simulink model "ofdm_txrx_supermimo"  (Simulink 7.1)
settings: Solver=VariableStepDiscrete  SolverMode=SingleTasking  StartTime=0.0  StopTime=simOnly_simLength  RelTol=1e-3
PostLoadFcn:
  | ofdm_rx_supermimo_init
InitFcn:
  | ofdm_rx_supermimo_init
StartFcn:
  | ofdm_rx_supermimo_init
values from neighbouring files (symbols this model uses):
  Action0_Config = 0* 31*2^18 + 0*2^24 + PHY_AUTORESPONSE_REQ_GOODHDR + PHY_AUTORESPONSE_REQ_GOODPKT + PHY_AUTORESPONSE_REQ_FLAGA    [ofdm_tx_supermimo_init.m]
  Action1_Config = 0* 62*2^18 + PHY_AUTORESPONSE_REQ_GOODHDR + PHY_AUTORESPONSE_REQ_GOODPKT    [ofdm_tx_supermimo_init.m]
  Action2_Config = 0    [ofdm_tx_supermimo_init.m]
  Action3_Config = 0    [ofdm_tx_supermimo_init.m]
  Action4_Config = 0    [ofdm_tx_supermimo_init.m]
  Action5_Config = 0    [ofdm_tx_supermimo_init.m]
  ActionID_AFTransmit = 31    [ofdm_tx_supermimo_init.m]
  ActionID_Disabled = 0    [ofdm_tx_supermimo_init.m]
  ActionID_SetFlagA = 62    [ofdm_tx_supermimo_init.m]
  ActionID_SetFlagB = 61    [ofdm_tx_supermimo_init.m]
  BER_RAM_init_size = 2048    [ofdm_tx_supermimo_init.m]
  CPLength = 16    [ofdm_tx_supermimo_init.m]
  longCorr_coef_i = [-4*real(LTS) 0]    [ofdm_rx_supermimo_init.m]
  longCorr_coef_q = [4*imag(LTS) 0]    [ofdm_rx_supermimo_init.m]
  Match0_Config = 0    [ofdm_tx_supermimo_init.m]
  Match1_Config = 0    [ofdm_tx_supermimo_init.m]
  Match2_Config = 0    [ofdm_tx_supermimo_init.m]
  Match3_Config = 0    [ofdm_tx_supermimo_init.m]
  Match4_Config = 0    [ofdm_tx_supermimo_init.m]
  Match5_Config = 0    [ofdm_tx_supermimo_init.m]
  Match6_Config = 0    [ofdm_tx_supermimo_init.m]
  Match7_Config = 0    [ofdm_tx_supermimo_init.m]
  max_CP_length = 16    [ofdm_tx_supermimo_init.m]
  max_num_baseRateSymbols = 31    [ofdm_tx_supermimo_init.m]
  max_num_subcarriers = 64    [ofdm_tx_supermimo_init.m]
  max_num_trainingSymbols = 15    [ofdm_tx_supermimo_init.m]
  max_numBytes = 16384    [ofdm_tx_supermimo_init.m]
  max_OFDM_symbols = 511    [ofdm_tx_supermimo_init.m]
  modConstellation_qam16 = .75*[1 3 -1 -3]./3    [ofdm_tx_supermimo_init.m]
  modConstellation_qam64 = 0.875*[3 1 5 7 -3 -1 -5 -7]./7    [ofdm_tx_supermimo_init.m]
  modConstellation_qpsk = [1 -1]./sqrt(2)    [ofdm_tx_supermimo_init.m]
  numBaseRateSymbols = ceil(numHeaderBytes / numBytes_BaseRateOFDMSymbol)    [ofdm_tx_supermimo_init.m]
  numSubcarriers = 64    [ofdm_tx_supermimo_init.m]
  numTrainingSymbols = 2    [ofdm_tx_supermimo_init.m]
  pilot_indicies = 7 + ( (64-21) * 2^8) + ( (64-7) * 2^16) + (21 * 2^24)    (= 356068103)    [ofdm_tx_supermimo_init.m]
  pilotValues = hex2dec('A57D') + (2^16 * hex2dec('5A82'))    [ofdm_tx_supermimo_init.m]
  pkt_crossCorr_thresh = 0.7    [ofdm_rx_supermimo_init.m]
  pkt_energy_thresh = 0    [ofdm_rx_supermimo_init.m]
  pktByteNums = numHeaderBytes +  				byteIndex_numPayloadBytes(1)*2^8 +  				byteIndex_numPayloadBytes(2)*2^16 +  				byteIndex_simpleDynModMasks * 2^24    [ofdm_rx_supermimo_init.m]
  PktDet_Delay = 58+32 +      Symbol_Timing_Offset*2^7 +      0*2^16 +      (117* 2^24)    (= 1962935642)    [ofdm_rx_supermimo_init.m]
  pktTiming_count_payloadStart = pktTiming_count_lsStart + pktTiming_ls_num*pktTiming_ls_length    [ofdm_rx_supermimo_init.m]
  preamble = preamble_scale*[shortsyms_10 longsyms_2]    [ofdm_tx_supermimo_init.m]
  preamble_ROM = preamble_I_ROM + preamble_Q_ROM*2^16    [ofdm_tx_supermimo_init.m]
  RAM_init_size = max_numBytes    (= 16384)    [ofdm_tx_supermimo_init.m]
  reg_AF_Tx_Blanking = (320+80) + ( (320+80+79)*2^16)    (= 31392144)    [ofdm_rx_supermimo_init.m]
  reg_AF_Tx_Scaling = 2944    [ofdm_rx_supermimo_init.m]
  reg_Interrupt_PktBuf_Control = reg_InterruptControl +  	1 * 2^16 +  	0 * 2^24    (= 65656)    [ofdm_rx_supermimo_init.m]
  reg_PilotCalcParams = (4 * 2^16) + (minPilotChanMag)    (= 262184.96)    [ofdm_rx_supermimo_init.m]
  regRx_chanEst_minMag = ((0.5)*2^15) + ((0.5 * 2^15)*2^16)    (= 1073758208)    [ofdm_rx_supermimo_init.m]
  regRx_coarseCalcCorrection = round((1.3e-3 - 1.2982e-3)*2^32)    [ofdm_rx_supermimo_init.m]
  regRx_pilotCalcCorrection = round(1.00115 * 2^31)    [ofdm_rx_supermimo_init.m]
  regRx_preCFOoptions = 0 * 2^0 +  	1 * 2^1 +  	0    (= 2)    [ofdm_rx_supermimo_init.m]
  rx_controlBits = 1 * 2  		 + 1 * 4  		 + 0 * 8  		 + 1 * 16 * rx_SISO_Mode  		 + 1 * 32  		 + 1 * 64  		 + 1 * 128  		 + 1 * 256  		 + 1 * 512  		 + 1 * 1024  		 + 1 * 4096  		 + 0 * 16384  		 + 0 * 32768  		 + 1 * 65536  		 + 0 * 131072  		 + 0 * 262144  		 + 1 * 524288  		 + 1 * 1048576 * tx_Alamouti_Mode  		 + 0 * 2097152           + 1 * 33554432  		 + 0 * 4194304  		 + 0 * 8388608  		 + 0 * 16777216  		 + 1 *  ...(+49 chars)    [ofdm_rx_supermimo_init.m]
  Rx_PktDet_LongCorr_Params = LongCorrCounterLoad + 0*2^8 + 0*2^16 + 255*2^24    (= 4278190325)    [ofdm_rx_supermimo_init.m]
  Rx_PktDet_LongCorrThresholds = 8000    [ofdm_rx_supermimo_init.m]
  rx_postEq_scaling = round(rxScaling*2^11) * (1 + 2^16)    [ofdm_rx_supermimo_init.m]
  RxDataScrambling_Seq = [40 198 78 63 82 173 102 245 48 111 172 115 147 230 216 93 72 65 62 2 205 242 122 90 128 83 105 97 73 10 5 252]    [ofdm_tx_supermimo_init.m]
  RxReg_FixedPktLen = 0    [ofdm_rx_supermimo_init.m]
  shortSymbol_time = shortSymbol_time(1:16).'    [ofdm_tx_supermimo_init.m]
  simOnly_numSamples = length(preamble)+( (numSubcarriers+CPLength)*(numTrainingSymbols + numBaseRateSymbols + numFullRateSymbols/2) )    [ofdm_tx_supermimo_init.m]
  train = [train train]    [ofdm_tx_supermimo_init.m]
  tx_controlBits = (antB_preambleShift * 2^4) + (2^1 * tx_Alamouti_Mode) +  	(2^0 * tx_SISO_Mode) + 4*0 + 8*1 + 0*256 + 512*0 + 1024*0 +  	0*hex2dec('1000') + 0*hex2dec('2000') + 0*hex2dec('4000') +  	1*hex2dec('8000') + 1*hex2dec('10000') + hex2dec('20000') +  	16*hex2dec('100000')    [ofdm_tx_supermimo_init.m]
  tx_delays = (50*2^0) + (0*2^8) + (30*2^12)    (= 122930)    [ofdm_tx_supermimo_init.m]
  tx_scaling = (3072) + (12288 * 2^16)    (= 805309440)    [ofdm_tx_supermimo_init.m]
  TxDataScrambling_Seq = [40 198 78 63 82 173 102 245 48 111 172 115 147 230 216 93 72 65 62 2 205 242 122 90 128 83 105 97 73 10 5 252]    [ofdm_tx_supermimo_init.m]
  txfilt_firpm = 0.95*txfilt_firpm./(max(txfilt_firpm))    [ofdm_tx_supermimo_init.m]
  txfilt_intfilt = [0 intfilt(4,4,.8)]    [ofdm_tx_supermimo_init.m]
  txReg_symbolCounts = (2^8 *numBaseRateSymbols ) + numTrainingSymbols    [ofdm_tx_supermimo_init.m]
  TxRx_FFTScaling = (3 * 2^0) +  	(2 * 2^2) +  	(1 * 2^4) +  	(1 * 2^6) +  	(1 * 2^8) +  	(0 * 2^10)    (= 347)    [ofdm_tx_supermimo_init.m]
undefined symbols (not defined in the file or in its folder): AntA, AntB, BaseRate, Down, gcbh, numBuffs, numBytes, Up, xlBlockLoadCallback, xlGetSimulinkPeriod, xlipgetpartsetting
libraries: xbsIndex_r4 x6035, simulink x4, dspsigops x4, commchan3 x2, dspsrcs4 x2, commrflib2 x2
(part 14 of 61)

== system ofdm_txrx_supermimo/OFDM Rx MIMO/Long Corr HP/C2 ==
  x : Inport  OutDataType=sfix(16)  OutScaling=2^0
  AddSub : xbsIndex_r4/AddSub  Orientation=down  mode=Addition  use_carryin=off  use_carryout=off  en=off  latency=0  precision=Full  arith_type=Unsigned  n_bits=8  bin_pt=2  quantization=Truncate  overflow=Wrap  dbl_ovrd=off  use_behavioral_HDL=on  pipelined=off  use_rpm=off  hw_selection=Fabric  block_type=addsub
      settings: xl_area=[3 0 0 4 0 0 0]  block_version=VER_STRING_GOES_HERE
  AddSub{1..7,9..14} (13 alike) : xbsIndex_r4/AddSub  Orientation=down  mode=Addition  use_carryin=off  use_carryout=off  en=off  latency=1  precision=Full  arith_type=Unsigned  n_bits=8  bin_pt=2  quantization=Truncate  overflow=Wrap  dbl_ovrd=off  use_behavioral_HDL=on  pipelined=off  use_rpm=off  hw_selection=Fabric  block_type=addsub
      AddSub1 settings: xl_area=[3 5 0 4 0 0 0]  block_version=VER_STRING_GOES_HERE
      AddSub10 settings: xl_area=[3 5 0 4 0 0 0]  block_version=VER_STRING_GOES_HERE
      AddSub11 settings: xl_area=[3 5 0 4 0 0 0]  block_version=VER_STRING_GOES_HERE
      AddSub12 settings: xl_area=[3 5 0 4 0 0 0]  block_version=VER_STRING_GOES_HERE
      AddSub13 settings: xl_area=[3 6 0 5 0 0 0]  block_version=VER_STRING_GOES_HERE
      AddSub14 settings: xl_area=[3 6 0 5 0 0 0]  block_version=VER_STRING_GOES_HERE
      AddSub2 settings: xl_area=[3 6 0 5 0 0 0]  block_version=VER_STRING_GOES_HERE
      AddSub3 settings: xl_area=[3 5 0 4 0 0 0]  block_version=VER_STRING_GOES_HERE
      AddSub4 settings: xl_area=[4 7 0 6 0 0 0]  block_version=VER_STRING_GOES_HERE
      AddSub5 settings: xl_area=[3 5 0 4 0 0 0]  block_version=VER_STRING_GOES_HERE
      AddSub6 settings: xl_area=[3 6 0 5 0 0 0]  block_version=VER_STRING_GOES_HERE
      AddSub7 settings: xl_area=[3 5 0 4 0 0 0]  block_version=VER_STRING_GOES_HERE
      AddSub9 settings: xl_area=[4 7 0 6 0 0 0]  block_version=VER_STRING_GOES_HERE
  AddSub8 : xbsIndex_r4/AddSub  mode=Addition  use_carryin=off  use_carryout=off  en=off  latency=1  precision=Full  arith_type=Unsigned  n_bits=8  bin_pt=2  quantization=Truncate  overflow=Wrap  dbl_ovrd=off  use_behavioral_HDL=on  pipelined=off  use_rpm=off  hw_selection=Fabric  block_type=addsub
      settings: xl_area=[4 8 0 7 0 0 0]  block_version=VER_STRING_GOES_HERE
  C1 : Subsystem  mask{coef2=h(1:N)}
      mask prompts: coef2 "Coefficients"
  C10 : Subsystem  mask{coef2=h(9*N+1:10*N)}
      mask prompts: coef2 "Coefficients"
  C11 : Subsystem  mask{coef2=h(10*N+1:11*N)}
      mask prompts: coef2 "Coefficients"
  C12 : Subsystem  mask{coef2=h(11*N+1:12*N)}
      mask prompts: coef2 "Coefficients"
  C13 : Subsystem  mask{coef2=h(12*N+1:13*N)}
      mask prompts: coef2 "Coefficients"
  C14 : Subsystem  mask{coef2=h(13*N+1:14*N)}
      mask prompts: coef2 "Coefficients"
  C15 : Subsystem  mask{coef2=h(14*N+1:15*N)}
      mask prompts: coef2 "Coefficients"
  C16 : Subsystem  mask{coef2=h(15*N+1:16*N)}
      mask prompts: coef2 "Coefficients"
  C2 : Subsystem  mask{coef2=h(N+1:2*N)}
      mask prompts: coef2 "Coefficients"
  C3 : Subsystem  mask{coef2=h(2*N+1:3*N)}
      mask prompts: coef2 "Coefficients"
  C4 : Subsystem  mask{coef2=h(3*N+1:4*N)}
      mask prompts: coef2 "Coefficients"
  C5 : Subsystem  mask{coef2=h(4*N+1:5*N)}
      mask prompts: coef2 "Coefficients"
  C6 : Subsystem  mask{coef2=h(5*N+1:6*N)}
      mask prompts: coef2 "Coefficients"
  C7 : Subsystem  mask{coef2=h(6*N+1:7*N)}
      mask prompts: coef2 "Coefficients"
  C8 : Subsystem  mask{coef2=h(7*N+1:8*N)}
      mask prompts: coef2 "Coefficients"
  C9 : Subsystem  mask{coef2=h(8*N+1:9*N)}
      mask prompts: coef2 "Coefficients"
  Delay{1,3..4,6,8,12..13,15} (8 alike) : xbsIndex_r4/Delay  Orientation=down  en=off  latency=N-1  dbl_ovrd=off  reg_retiming=off  block_type=delay
      settings: infoedit=Hardware notes: A delay line is a chain, each link of which is an SRL16 followed by a flip-flop. If register retiming is enabled, the delay line is a chain of flip-flops.  xl_area=[2 4 0 4 0 0 0]  block_version=VER_STRING_GOES_HERE
  Delay10 : xbsIndex_r4/Delay  Orientation=down  en=off  latency=((N-1)*12)-1  dbl_ovrd=off  reg_retiming=off  block_type=delay
      settings: infoedit=Hardware notes: A delay line is a chain, each link of which is an SRL16 followed by a flip-flop. If register retiming is enabled, the delay line is a chain of flip-flops.  xl_area=[9 15 0 15 0 0 0]  block_version=VER_STRING_GOES_HERE
  Delay11 : xbsIndex_r4/Delay  Orientation=down  en=off  latency=((N-1)*14)-1  dbl_ovrd=off  reg_retiming=off  block_type=delay
      settings: infoedit=Hardware notes: A delay line is a chain, each link of which is an SRL16 followed by a flip-flop. If register retiming is enabled, the delay line is a chain of flip-flops.  xl_area=[9 15 0 15 0 0 0]  block_version=VER_STRING_GOES_HERE
  Delay14 : xbsIndex_r4/Delay  Orientation=down  en=off  latency=((N-1)*2)-1  dbl_ovrd=off  reg_retiming=off  block_type=delay
      settings: infoedit=Hardware notes: A delay line is a chain, each link of which is an SRL16 followed by a flip-flop. If register retiming is enabled, the delay line is a chain of flip-flops.  xl_area=[3 5 0 5 0 0 0]  block_version=VER_STRING_GOES_HERE
  Delay2 : xbsIndex_r4/Delay  Orientation=down  en=off  latency=((N-1)*4)-1  dbl_ovrd=off  reg_retiming=off  block_type=delay
      settings: infoedit=Hardware notes: A delay line is a chain, each link of which is an SRL16 followed by a flip-flop. If register retiming is enabled, the delay line is a chain of flip-flops.  xl_area=[3 5 0 5 0 0 0]  block_version=VER_STRING_GOES_HERE
  Delay5 : xbsIndex_r4/Delay  Orientation=down  en=off  latency=((N-1)*6)-1  dbl_ovrd=off  reg_retiming=off  block_type=delay
      settings: infoedit=Hardware notes: A delay line is a chain, each link of which is an SRL16 followed by a flip-flop. If register retiming is enabled, the delay line is a chain of flip-flops.  xl_area=[6 10 0 10 0 0 0]  block_version=VER_STRING_GOES_HERE
  Delay7 : xbsIndex_r4/Delay  Orientation=down  en=off  latency=((N-1)*8)-1  dbl_ovrd=off  reg_retiming=off  block_type=delay
      settings: infoedit=Hardware notes: A delay line is a chain, each link of which is an SRL16 followed by a flip-flop. If register retiming is enabled, the delay line is a chain of flip-flops.  xl_area=[6 10 0 10 0 0 0]  block_version=VER_STRING_GOES_HERE
  Delay9 : xbsIndex_r4/Delay  Orientation=down  en=off  latency=((N-1)*10)-1  dbl_ovrd=off  reg_retiming=off  block_type=delay
      settings: infoedit=Hardware notes: A delay line is a chain, each link of which is an SRL16 followed by a flip-flop. If register retiming is enabled, the delay line is a chain of flip-flops.  xl_area=[9 15 0 15 0 0 0]  block_version=VER_STRING_GOES_HERE
  FSM : Subsystem
  Gateway Out6 : xbsIndex_r4/Gateway Out  hdl_port=off  timing_constraint=None  locs_specified=off  LOCs={}  block_type=gatewayout
      settings: infoedit="Gateway out block.  Converts Xilinx fixed point inputs into ouputs of type Simulink integer, double, or fixed point.<P><P>Hardware notes:  In hardware these blocks become top level output ports or are discarded, depending on how they are configured."  xl_area=[0 0 0 0 0 0 0]  block_version=VER_STRING_GOES_HERE
  Gateway Out7 : xbsIndex_r4/Gateway Out  hdl_port=off  timing_constraint=None  locs_specified=off  LOCs={}  block_type=gatewayout
      settings: infoedit="Gateway out block.  Converts Xilinx fixed point inputs into ouputs of type Simulink integer, double, or fixed point.<P><P>Hardware notes:  In hardware these blocks become top level output ports or are discarded, depending on how they are configured."  xl_area=[0 0 0 0 0 0 0]  block_version=VER_STRING_GOES_HERE
  Gateway Out8 : xbsIndex_r4/Gateway Out  hdl_port=off  timing_constraint=None  locs_specified=off  LOCs={}  block_type=gatewayout
      settings: infoedit="Gateway out block.  Converts Xilinx fixed point inputs into ouputs of type Simulink integer, double, or fixed point.<P><P>Hardware notes:  In hardware these blocks become top level output ports or are discarded, depending on how they are configured."  xl_area=[0 0 0 0 0 0 0]  block_version=VER_STRING_GOES_HERE
  Long Corr Scope2 : Scope  NumInputPorts=3
      settings: YMin=-0.35~-0.175~0  YMax=0.35~0.225~1300  SaveName=ScopeData8
  y : Outport  OutDataType=sfix(16)  OutScaling=2^0
  wires:
  Gateway Out8/1 -> Long Corr Scope2/3
  Gateway Out7/1 -> Long Corr Scope2/2
  Gateway Out6/1 -> Long Corr Scope2/1
  AddSub14/1 -> Gateway Out6/1, AddSub9/2
  Delay11/1 -> AddSub14/2
  Delay10/1 -> AddSub14/1
  AddSub11/1 -> Delay11/1
  AddSub10/1 -> Delay10/1
  Delay15/1 -> AddSub11/2
  Delay12/1 -> AddSub10/2
  C16/1(yn) -> Delay15/1
  C14/1(yn) -> Delay12/1
  C15/1(yn) -> AddSub11/1
  C15/2(xnz) -> C16/1(xn)
  C14/2(xnz) -> C15/1(xn)
  C13/2(xnz) -> C14/1(xn)
  x/1 -> C1/1(xn)
  C2/1(yn) -> Delay3/1
  C1/2(xnz) -> C2/1(xn)
  FSM/1(Data Addr) -> C2/2(DAddr), C3/2(DAddr), C4/2(DAddr), C5/2(DAddr), C6/2(DAddr), C7/2(DAddr), C8/2(DAddr), C1/2(DAddr), C9/2(DAddr), C10/2(DAddr), C11/2(DAddr), C15/2(DAddr), C16/2(DAddr), C14/2(DAddr), C13/2(DAddr), C12/2(DAddr)
  FSM/2(Coef Addr) -> C2/3(CAddr), C3/3(CAddr), C4/3(CAddr), C5/3(CAddr), C6/3(CAddr), C7/3(CAddr), C8/3(CAddr), C1/3(CAddr), C9/3(CAddr), C10/3(CAddr), C11/3(CAddr), C15/3(CAddr), C16/3(CAddr), C14/3(CAddr), C13/3(CAddr), C12/3(CAddr)
  FSM/3(load) -> C2/4(LD), C3/4(LD), C4/4(LD), C5/4(LD), C6/4(LD), C7/4(LD), C8/4(LD), C1/4(LD), C9/4(LD), C10/4(LD), C11/4(LD), C15/4(LD), C16/4(LD), C14/4(LD), C13/4(LD), C12/4(LD)
  C2/2(xnz) -> C3/1(xn)
  C3/2(xnz) -> C4/1(xn)
  C3/1(yn) -> AddSub12/1
  C4/2(xnz) -> C5/1(xn)
  C4/1(yn) -> Delay13/1
  C5/2(xnz) -> C6/1(xn)
  C6/2(xnz) -> C7/1(xn)
  [xnz] -> C9/1(xn)
  C9/2(xnz) -> C10/1(xn)
  C10/2(xnz) -> C11/1(xn)
  C11/2(xnz) -> C12/1(xn)
  C12/2(xnz) -> C13/1(xn)
  Delay13/1 -> AddSub12/2
  AddSub12/1 -> Delay14/1
  Delay3/1 -> AddSub/2
  C1/1(yn) -> AddSub/1
  C5/1(yn) -> AddSub1/1
  Delay1/1 -> AddSub1/2
  AddSub1/1 -> Delay2/1
  C6/1(yn) -> Delay1/1
  C7/2(xnz) -> C8/1(xn)
  Delay4/1 -> AddSub3/2
  AddSub3/1 -> Delay5/1
  C7/1(yn) -> AddSub3/1
  C8/1(yn) -> Delay4/1
  C9/1(yn) -> AddSub5/1
  Delay6/1 -> AddSub5/2
  AddSub5/1 -> Delay7/1
  C10/1(yn) -> Delay6/1
  C8/2(xnz) -> [xnz]
  Delay14/1 -> AddSub13/2
  AddSub/1 -> AddSub13/1
  Delay2/1 -> AddSub2/1
  Delay5/1 -> AddSub2/2
  AddSub13/1 -> AddSub4/1
  AddSub2/1 -> AddSub4/2
  AddSub4/1 -> AddSub8/1
  C11/1(yn) -> AddSub7/1
  Delay8/1 -> AddSub7/2
  AddSub7/1 -> Delay9/1
  C12/1(yn) -> Delay8/1
  Delay7/1 -> AddSub6/1
  Delay9/1 -> AddSub6/2
  AddSub8/1 -> y/1
  C13/1(yn) -> AddSub10/1
  AddSub6/1 -> Gateway Out7/1, AddSub9/1
  AddSub9/1 -> AddSub8/2, Gateway Out8/1

== system ofdm_txrx_supermimo/OFDM Rx MIMO/Long Corr HP/C3 ==
  x : Inport  OutDataType=sfix(16)  OutScaling=2^0
  AddSub : xbsIndex_r4/AddSub  Orientation=down  mode=Addition  use_carryin=off  use_carryout=off  en=off  latency=0  precision=Full  arith_type=Unsigned  n_bits=8  bin_pt=2  quantization=Truncate  overflow=Wrap  dbl_ovrd=off  use_behavioral_HDL=on  pipelined=off  use_rpm=off  hw_selection=Fabric  block_type=addsub
      settings: xl_area=[3 0 0 4 0 0 0]  block_version=VER_STRING_GOES_HERE
  AddSub{1..7,9..14} (13 alike) : xbsIndex_r4/AddSub  Orientation=down  mode=Addition  use_carryin=off  use_carryout=off  en=off  latency=1  precision=Full  arith_type=Unsigned  n_bits=8  bin_pt=2  quantization=Truncate  overflow=Wrap  dbl_ovrd=off  use_behavioral_HDL=on  pipelined=off  use_rpm=off  hw_selection=Fabric  block_type=addsub
      AddSub1 settings: xl_area=[3 5 0 4 0 0 0]  block_version=VER_STRING_GOES_HERE
      AddSub10 settings: xl_area=[3 5 0 4 0 0 0]  block_version=VER_STRING_GOES_HERE
      AddSub11 settings: xl_area=[3 5 0 4 0 0 0]  block_version=VER_STRING_GOES_HERE
      AddSub12 settings: xl_area=[3 5 0 4 0 0 0]  block_version=VER_STRING_GOES_HERE
      AddSub13 settings: xl_area=[3 6 0 5 0 0 0]  block_version=VER_STRING_GOES_HERE
      AddSub14 settings: xl_area=[3 6 0 5 0 0 0]  block_version=VER_STRING_GOES_HERE
      AddSub2 settings: xl_area=[3 6 0 5 0 0 0]  block_version=VER_STRING_GOES_HERE
      AddSub3 settings: xl_area=[3 5 0 4 0 0 0]  block_version=VER_STRING_GOES_HERE
      AddSub4 settings: xl_area=[4 7 0 6 0 0 0]  block_version=VER_STRING_GOES_HERE
      AddSub5 settings: xl_area=[3 5 0 4 0 0 0]  block_version=VER_STRING_GOES_HERE
      AddSub6 settings: xl_area=[3 6 0 5 0 0 0]  block_version=VER_STRING_GOES_HERE
      AddSub7 settings: xl_area=[3 5 0 4 0 0 0]  block_version=VER_STRING_GOES_HERE
      AddSub9 settings: xl_area=[4 7 0 6 0 0 0]  block_version=VER_STRING_GOES_HERE
  AddSub8 : xbsIndex_r4/AddSub  mode=Addition  use_carryin=off  use_carryout=off  en=off  latency=1  precision=Full  arith_type=Unsigned  n_bits=8  bin_pt=2  quantization=Truncate  overflow=Wrap  dbl_ovrd=off  use_behavioral_HDL=on  pipelined=off  use_rpm=off  hw_selection=Fabric  block_type=addsub
      settings: xl_area=[4 8 0 7 0 0 0]  block_version=VER_STRING_GOES_HERE
  C1 : Subsystem  mask{coef2=h(1:N)}
      mask prompts: coef2 "Coefficients"
  C10 : Subsystem  mask{coef2=h(9*N+1:10*N)}
      mask prompts: coef2 "Coefficients"
  C11 : Subsystem  mask{coef2=h(10*N+1:11*N)}
      mask prompts: coef2 "Coefficients"
  C12 : Subsystem  mask{coef2=h(11*N+1:12*N)}
      mask prompts: coef2 "Coefficients"
  C13 : Subsystem  mask{coef2=h(12*N+1:13*N)}
      mask prompts: coef2 "Coefficients"
  C14 : Subsystem  mask{coef2=h(13*N+1:14*N)}
      mask prompts: coef2 "Coefficients"
  C15 : Subsystem  mask{coef2=h(14*N+1:15*N)}
      mask prompts: coef2 "Coefficients"
  C16 : Subsystem  mask{coef2=h(15*N+1:16*N)}
      mask prompts: coef2 "Coefficients"
  C2 : Subsystem  mask{coef2=h(N+1:2*N)}
      mask prompts: coef2 "Coefficients"
  C3 : Subsystem  mask{coef2=h(2*N+1:3*N)}
      mask prompts: coef2 "Coefficients"
  C4 : Subsystem  mask{coef2=h(3*N+1:4*N)}
      mask prompts: coef2 "Coefficients"
  C5 : Subsystem  mask{coef2=h(4*N+1:5*N)}
      mask prompts: coef2 "Coefficients"
  C6 : Subsystem  mask{coef2=h(5*N+1:6*N)}
      mask prompts: coef2 "Coefficients"
  C7 : Subsystem  mask{coef2=h(6*N+1:7*N)}
      mask prompts: coef2 "Coefficients"
  C8 : Subsystem  mask{coef2=h(7*N+1:8*N)}
      mask prompts: coef2 "Coefficients"
  C9 : Subsystem  mask{coef2=h(8*N+1:9*N)}
      mask prompts: coef2 "Coefficients"
  Delay{1,3..4,6,8,12..13,15} (8 alike) : xbsIndex_r4/Delay  Orientation=down  en=off  latency=N-1  dbl_ovrd=off  reg_retiming=off  block_type=delay
      settings: infoedit=Hardware notes: A delay line is a chain, each link of which is an SRL16 followed by a flip-flop. If register retiming is enabled, the delay line is a chain of flip-flops.  xl_area=[2 4 0 4 0 0 0]  block_version=VER_STRING_GOES_HERE
  Delay10 : xbsIndex_r4/Delay  Orientation=down  en=off  latency=((N-1)*12)-1  dbl_ovrd=off  reg_retiming=off  block_type=delay
      settings: infoedit=Hardware notes: A delay line is a chain, each link of which is an SRL16 followed by a flip-flop. If register retiming is enabled, the delay line is a chain of flip-flops.  xl_area=[9 15 0 15 0 0 0]  block_version=VER_STRING_GOES_HERE
  Delay11 : xbsIndex_r4/Delay  Orientation=down  en=off  latency=((N-1)*14)-1  dbl_ovrd=off  reg_retiming=off  block_type=delay
      settings: infoedit=Hardware notes: A delay line is a chain, each link of which is an SRL16 followed by a flip-flop. If register retiming is enabled, the delay line is a chain of flip-flops.  xl_area=[9 15 0 15 0 0 0]  block_version=VER_STRING_GOES_HERE
  Delay14 : xbsIndex_r4/Delay  Orientation=down  en=off  latency=((N-1)*2)-1  dbl_ovrd=off  reg_retiming=off  block_type=delay
      settings: infoedit=Hardware notes: A delay line is a chain, each link of which is an SRL16 followed by a flip-flop. If register retiming is enabled, the delay line is a chain of flip-flops.  xl_area=[3 5 0 5 0 0 0]  block_version=VER_STRING_GOES_HERE
  Delay2 : xbsIndex_r4/Delay  Orientation=down  en=off  latency=((N-1)*4)-1  dbl_ovrd=off  reg_retiming=off  block_type=delay
      settings: infoedit=Hardware notes: A delay line is a chain, each link of which is an SRL16 followed by a flip-flop. If register retiming is enabled, the delay line is a chain of flip-flops.  xl_area=[3 5 0 5 0 0 0]  block_version=VER_STRING_GOES_HERE
  Delay5 : xbsIndex_r4/Delay  Orientation=down  en=off  latency=((N-1)*6)-1  dbl_ovrd=off  reg_retiming=off  block_type=delay
      settings: infoedit=Hardware notes: A delay line is a chain, each link of which is an SRL16 followed by a flip-flop. If register retiming is enabled, the delay line is a chain of flip-flops.  xl_area=[6 10 0 10 0 0 0]  block_version=VER_STRING_GOES_HERE
  Delay7 : xbsIndex_r4/Delay  Orientation=down  en=off  latency=((N-1)*8)-1  dbl_ovrd=off  reg_retiming=off  block_type=delay
      settings: infoedit=Hardware notes: A delay line is a chain, each link of which is an SRL16 followed by a flip-flop. If register retiming is enabled, the delay line is a chain of flip-flops.  xl_area=[6 10 0 10 0 0 0]  block_version=VER_STRING_GOES_HERE
  Delay9 : xbsIndex_r4/Delay  Orientation=down  en=off  latency=((N-1)*10)-1  dbl_ovrd=off  reg_retiming=off  block_type=delay
      settings: infoedit=Hardware notes: A delay line is a chain, each link of which is an SRL16 followed by a flip-flop. If register retiming is enabled, the delay line is a chain of flip-flops.  xl_area=[9 15 0 15 0 0 0]  block_version=VER_STRING_GOES_HERE
  FSM : Subsystem
  Gateway Out6 : xbsIndex_r4/Gateway Out  hdl_port=off  timing_constraint=None  locs_specified=off  LOCs={}  block_type=gatewayout
      settings: infoedit="Gateway out block.  Converts Xilinx fixed point inputs into ouputs of type Simulink integer, double, or fixed point.<P><P>Hardware notes:  In hardware these blocks become top level output ports or are discarded, depending on how they are configured."  xl_area=[0 0 0 0 0 0 0]  block_version=VER_STRING_GOES_HERE
  Gateway Out7 : xbsIndex_r4/Gateway Out  hdl_port=off  timing_constraint=None  locs_specified=off  LOCs={}  block_type=gatewayout
      settings: infoedit="Gateway out block.  Converts Xilinx fixed point inputs into ouputs of type Simulink integer, double, or fixed point.<P><P>Hardware notes:  In hardware these blocks become top level output ports or are discarded, depending on how they are configured."  xl_area=[0 0 0 0 0 0 0]  block_version=VER_STRING_GOES_HERE
  Gateway Out8 : xbsIndex_r4/Gateway Out  hdl_port=off  timing_constraint=None  locs_specified=off  LOCs={}  block_type=gatewayout
      settings: infoedit="Gateway out block.  Converts Xilinx fixed point inputs into ouputs of type Simulink integer, double, or fixed point.<P><P>Hardware notes:  In hardware these blocks become top level output ports or are discarded, depending on how they are configured."  xl_area=[0 0 0 0 0 0 0]  block_version=VER_STRING_GOES_HERE
  Long Corr Scope2 : Scope  NumInputPorts=3
      settings: YMin=-0.35~-0.175~0  YMax=0.35~0.225~1300  SaveName=ScopeData8
  y : Outport  OutDataType=sfix(16)  OutScaling=2^0
  wires:
  AddSub9/1 -> Gateway Out8/1, AddSub8/2
  AddSub6/1 -> AddSub9/1, Gateway Out7/1
  C13/1(yn) -> AddSub10/1
  AddSub8/1 -> y/1
  Delay9/1 -> AddSub6/2
  Delay7/1 -> AddSub6/1
  C12/1(yn) -> Delay8/1
  AddSub7/1 -> Delay9/1
  Delay8/1 -> AddSub7/2
  C11/1(yn) -> AddSub7/1
  AddSub4/1 -> AddSub8/1
  AddSub2/1 -> AddSub4/2
  AddSub13/1 -> AddSub4/1
  Delay5/1 -> AddSub2/2
  Delay2/1 -> AddSub2/1
  AddSub/1 -> AddSub13/1
  Delay14/1 -> AddSub13/2
  C8/2(xnz) -> [xnz]
  C10/1(yn) -> Delay6/1
  AddSub5/1 -> Delay7/1
  Delay6/1 -> AddSub5/2
  C9/1(yn) -> AddSub5/1
  C8/1(yn) -> Delay4/1
  C7/1(yn) -> AddSub3/1
  AddSub3/1 -> Delay5/1
  Delay4/1 -> AddSub3/2
  C7/2(xnz) -> C8/1(xn)
  C6/1(yn) -> Delay1/1
  AddSub1/1 -> Delay2/1
  Delay1/1 -> AddSub1/2
  C5/1(yn) -> AddSub1/1
  C1/1(yn) -> AddSub/1
  Delay3/1 -> AddSub/2
  AddSub12/1 -> Delay14/1
  Delay13/1 -> AddSub12/2
  C12/2(xnz) -> C13/1(xn)
  C11/2(xnz) -> C12/1(xn)
  C10/2(xnz) -> C11/1(xn)
  C9/2(xnz) -> C10/1(xn)
  [xnz] -> C9/1(xn)
  C6/2(xnz) -> C7/1(xn)
  C5/2(xnz) -> C6/1(xn)
  C4/1(yn) -> Delay13/1
  C4/2(xnz) -> C5/1(xn)
  C3/1(yn) -> AddSub12/1
  C3/2(xnz) -> C4/1(xn)
  C2/2(xnz) -> C3/1(xn)
  FSM/3(load) -> C12/4(LD), C13/4(LD), C14/4(LD), C16/4(LD), C15/4(LD), C11/4(LD), C10/4(LD), C9/4(LD), C1/4(LD), C8/4(LD), C7/4(LD), C6/4(LD), C5/4(LD), C4/4(LD), C3/4(LD), C2/4(LD)
  FSM/2(Coef Addr) -> C12/3(CAddr), C13/3(CAddr), C14/3(CAddr), C16/3(CAddr), C15/3(CAddr), C11/3(CAddr), C10/3(CAddr), C9/3(CAddr), C1/3(CAddr), C8/3(CAddr), C7/3(CAddr), C6/3(CAddr), C5/3(CAddr), C4/3(CAddr), C3/3(CAddr), C2/3(CAddr)
  FSM/1(Data Addr) -> C12/2(DAddr), C13/2(DAddr), C14/2(DAddr), C16/2(DAddr), C15/2(DAddr), C11/2(DAddr), C10/2(DAddr), C9/2(DAddr), C1/2(DAddr), C8/2(DAddr), C7/2(DAddr), C6/2(DAddr), C5/2(DAddr), C4/2(DAddr), C3/2(DAddr), C2/2(DAddr)
  C1/2(xnz) -> C2/1(xn)
  C2/1(yn) -> Delay3/1
  x/1 -> C1/1(xn)
  C13/2(xnz) -> C14/1(xn)
  C14/2(xnz) -> C15/1(xn)
  C15/2(xnz) -> C16/1(xn)
  C15/1(yn) -> AddSub11/1
  C14/1(yn) -> Delay12/1
  C16/1(yn) -> Delay15/1
  Delay12/1 -> AddSub10/2
  Delay15/1 -> AddSub11/2
  AddSub10/1 -> Delay10/1
  AddSub11/1 -> Delay11/1
  Delay10/1 -> AddSub14/1
  Delay11/1 -> AddSub14/2
  AddSub14/1 -> AddSub9/2, Gateway Out6/1
  Gateway Out6/1 -> Long Corr Scope2/1
  Gateway Out7/1 -> Long Corr Scope2/2
  Gateway Out8/1 -> Long Corr Scope2/3

== system ofdm_txrx_supermimo/OFDM Rx MIMO/Long Corr HP/C4 ==
  x : Inport  OutDataType=sfix(16)  OutScaling=2^0
  AddSub : xbsIndex_r4/AddSub  Orientation=down  mode=Addition  use_carryin=off  use_carryout=off  en=off  latency=0  precision=Full  arith_type=Unsigned  n_bits=8  bin_pt=2  quantization=Truncate  overflow=Wrap  dbl_ovrd=off  use_behavioral_HDL=on  pipelined=off  use_rpm=off  hw_selection=Fabric  block_type=addsub
      settings: xl_area=[3 0 0 4 0 0 0]  block_version=VER_STRING_GOES_HERE
  AddSub{1..7,9..14} (13 alike) : xbsIndex_r4/AddSub  Orientation=down  mode=Addition  use_carryin=off  use_carryout=off  en=off  latency=1  precision=Full  arith_type=Unsigned  n_bits=8  bin_pt=2  quantization=Truncate  overflow=Wrap  dbl_ovrd=off  use_behavioral_HDL=on  pipelined=off  use_rpm=off  hw_selection=Fabric  block_type=addsub
      AddSub1 settings: xl_area=[3 5 0 4 0 0 0]  block_version=VER_STRING_GOES_HERE
      AddSub10 settings: xl_area=[3 5 0 4 0 0 0]  block_version=VER_STRING_GOES_HERE
      AddSub11 settings: xl_area=[3 5 0 4 0 0 0]  block_version=VER_STRING_GOES_HERE
      AddSub12 settings: xl_area=[3 5 0 4 0 0 0]  block_version=VER_STRING_GOES_HERE
      AddSub13 settings: xl_area=[3 6 0 5 0 0 0]  block_version=VER_STRING_GOES_HERE
      AddSub14 settings: xl_area=[3 6 0 5 0 0 0]  block_version=VER_STRING_GOES_HERE
      AddSub2 settings: xl_area=[3 6 0 5 0 0 0]  block_version=VER_STRING_GOES_HERE
      AddSub3 settings: xl_area=[3 5 0 4 0 0 0]  block_version=VER_STRING_GOES_HERE
      AddSub4 settings: xl_area=[4 7 0 6 0 0 0]  block_version=VER_STRING_GOES_HERE
      AddSub5 settings: xl_area=[3 5 0 4 0 0 0]  block_version=VER_STRING_GOES_HERE
      AddSub6 settings: xl_area=[3 6 0 5 0 0 0]  block_version=VER_STRING_GOES_HERE
      AddSub7 settings: xl_area=[3 5 0 4 0 0 0]  block_version=VER_STRING_GOES_HERE
      AddSub9 settings: xl_area=[4 7 0 6 0 0 0]  block_version=VER_STRING_GOES_HERE
  AddSub8 : xbsIndex_r4/AddSub  mode=Addition  use_carryin=off  use_carryout=off  en=off  latency=1  precision=Full  arith_type=Unsigned  n_bits=8  bin_pt=2  quantization=Truncate  overflow=Wrap  dbl_ovrd=off  use_behavioral_HDL=on  pipelined=off  use_rpm=off  hw_selection=Fabric  block_type=addsub
      settings: xl_area=[4 8 0 7 0 0 0]  block_version=VER_STRING_GOES_HERE
  C1 : Subsystem  mask{coef2=h(1:N)}
      mask prompts: coef2 "Coefficients"
  C10 : Subsystem  mask{coef2=h(9*N+1:10*N)}
      mask prompts: coef2 "Coefficients"
  C11 : Subsystem  mask{coef2=h(10*N+1:11*N)}
      mask prompts: coef2 "Coefficients"
  C12 : Subsystem  mask{coef2=h(11*N+1:12*N)}
      mask prompts: coef2 "Coefficients"
  C13 : Subsystem  mask{coef2=h(12*N+1:13*N)}
      mask prompts: coef2 "Coefficients"
  C14 : Subsystem  mask{coef2=h(13*N+1:14*N)}
      mask prompts: coef2 "Coefficients"
  C15 : Subsystem  mask{coef2=h(14*N+1:15*N)}
      mask prompts: coef2 "Coefficients"
  C16 : Subsystem  mask{coef2=h(15*N+1:16*N)}
      mask prompts: coef2 "Coefficients"
  C2 : Subsystem  mask{coef2=h(N+1:2*N)}
      mask prompts: coef2 "Coefficients"
  C3 : Subsystem  mask{coef2=h(2*N+1:3*N)}
      mask prompts: coef2 "Coefficients"
  C4 : Subsystem  mask{coef2=h(3*N+1:4*N)}
      mask prompts: coef2 "Coefficients"
  C5 : Subsystem  mask{coef2=h(4*N+1:5*N)}
      mask prompts: coef2 "Coefficients"
  C6 : Subsystem  mask{coef2=h(5*N+1:6*N)}
      mask prompts: coef2 "Coefficients"
  C7 : Subsystem  mask{coef2=h(6*N+1:7*N)}
      mask prompts: coef2 "Coefficients"
  C8 : Subsystem  mask{coef2=h(7*N+1:8*N)}
      mask prompts: coef2 "Coefficients"
  C9 : Subsystem  mask{coef2=h(8*N+1:9*N)}
      mask prompts: coef2 "Coefficients"
  Delay{1,3..4,6,8,12..13,15} (8 alike) : xbsIndex_r4/Delay  Orientation=down  en=off  latency=N-1  dbl_ovrd=off  reg_retiming=off  block_type=delay
      settings: infoedit=Hardware notes: A delay line is a chain, each link of which is an SRL16 followed by a flip-flop. If register retiming is enabled, the delay line is a chain of flip-flops.  xl_area=[2 4 0 4 0 0 0]  block_version=VER_STRING_GOES_HERE
  Delay10 : xbsIndex_r4/Delay  Orientation=down  en=off  latency=((N-1)*12)-1  dbl_ovrd=off  reg_retiming=off  block_type=delay
      settings: infoedit=Hardware notes: A delay line is a chain, each link of which is an SRL16 followed by a flip-flop. If register retiming is enabled, the delay line is a chain of flip-flops.  xl_area=[9 15 0 15 0 0 0]  block_version=VER_STRING_GOES_HERE
  Delay11 : xbsIndex_r4/Delay  Orientation=down  en=off  latency=((N-1)*14)-1  dbl_ovrd=off  reg_retiming=off  block_type=delay
      settings: infoedit=Hardware notes: A delay line is a chain, each link of which is an SRL16 followed by a flip-flop. If register retiming is enabled, the delay line is a chain of flip-flops.  xl_area=[9 15 0 15 0 0 0]  block_version=VER_STRING_GOES_HERE
  Delay14 : xbsIndex_r4/Delay  Orientation=down  en=off  latency=((N-1)*2)-1  dbl_ovrd=off  reg_retiming=off  block_type=delay
      settings: infoedit=Hardware notes: A delay line is a chain, each link of which is an SRL16 followed by a flip-flop. If register retiming is enabled, the delay line is a chain of flip-flops.  xl_area=[3 5 0 5 0 0 0]  block_version=VER_STRING_GOES_HERE
  Delay2 : xbsIndex_r4/Delay  Orientation=down  en=off  latency=((N-1)*4)-1  dbl_ovrd=off  reg_retiming=off  block_type=delay
      settings: infoedit=Hardware notes: A delay line is a chain, each link of which is an SRL16 followed by a flip-flop. If register retiming is enabled, the delay line is a chain of flip-flops.  xl_area=[3 5 0 5 0 0 0]  block_version=VER_STRING_GOES_HERE
  Delay5 : xbsIndex_r4/Delay  Orientation=down  en=off  latency=((N-1)*6)-1  dbl_ovrd=off  reg_retiming=off  block_type=delay
      settings: infoedit=Hardware notes: A delay line is a chain, each link of which is an SRL16 followed by a flip-flop. If register retiming is enabled, the delay line is a chain of flip-flops.  xl_area=[6 10 0 10 0 0 0]  block_version=VER_STRING_GOES_HERE
  Delay7 : xbsIndex_r4/Delay  Orientation=down  en=off  latency=((N-1)*8)-1  dbl_ovrd=off  reg_retiming=off  block_type=delay
      settings: infoedit=Hardware notes: A delay line is a chain, each link of which is an SRL16 followed by a flip-flop. If register retiming is enabled, the delay line is a chain of flip-flops.  xl_area=[6 10 0 10 0 0 0]  block_version=VER_STRING_GOES_HERE
  Delay9 : xbsIndex_r4/Delay  Orientation=down  en=off  latency=((N-1)*10)-1  dbl_ovrd=off  reg_retiming=off  block_type=delay
      settings: infoedit=Hardware notes: A delay line is a chain, each link of which is an SRL16 followed by a flip-flop. If register retiming is enabled, the delay line is a chain of flip-flops.  xl_area=[9 15 0 15 0 0 0]  block_version=VER_STRING_GOES_HERE
  FSM : Subsystem
  Gateway Out6 : xbsIndex_r4/Gateway Out  hdl_port=off  timing_constraint=None  locs_specified=off  LOCs={}  block_type=gatewayout
      settings: infoedit="Gateway out block.  Converts Xilinx fixed point inputs into ouputs of type Simulink integer, double, or fixed point.<P><P>Hardware notes:  In hardware these blocks become top level output ports or are discarded, depending on how they are configured."  xl_area=[0 0 0 0 0 0 0]  block_version=VER_STRING_GOES_HERE
  Gateway Out7 : xbsIndex_r4/Gateway Out  hdl_port=off  timing_constraint=None  locs_specified=off  LOCs={}  block_type=gatewayout
      settings: infoedit="Gateway out block.  Converts Xilinx fixed point inputs into ouputs of type Simulink integer, double, or fixed point.<P><P>Hardware notes:  In hardware these blocks become top level output ports or are discarded, depending on how they are configured."  xl_area=[0 0 0 0 0 0 0]  block_version=VER_STRING_GOES_HERE
  Gateway Out8 : xbsIndex_r4/Gateway Out  hdl_port=off  timing_constraint=None  locs_specified=off  LOCs={}  block_type=gatewayout
      settings: infoedit="Gateway out block.  Converts Xilinx fixed point inputs into ouputs of type Simulink integer, double, or fixed point.<P><P>Hardware notes:  In hardware these blocks become top level output ports or are discarded, depending on how they are configured."  xl_area=[0 0 0 0 0 0 0]  block_version=VER_STRING_GOES_HERE
  Long Corr Scope2 : Scope  NumInputPorts=3
      settings: YMin=-0.35~-0.175~0  YMax=0.35~0.225~1300  SaveName=ScopeData8
  y : Outport  OutDataType=sfix(16)  OutScaling=2^0
  wires:
  AddSub9/1 -> Gateway Out8/1, AddSub8/2
  AddSub6/1 -> AddSub9/1, Gateway Out7/1
  C13/1(yn) -> AddSub10/1
  AddSub8/1 -> y/1
  Delay9/1 -> AddSub6/2
  Delay7/1 -> AddSub6/1
  C12/1(yn) -> Delay8/1
  AddSub7/1 -> Delay9/1
  Delay8/1 -> AddSub7/2
  C11/1(yn) -> AddSub7/1
  AddSub4/1 -> AddSub8/1
  AddSub2/1 -> AddSub4/2
  AddSub13/1 -> AddSub4/1
  Delay5/1 -> AddSub2/2
  Delay2/1 -> AddSub2/1
  AddSub/1 -> AddSub13/1
  Delay14/1 -> AddSub13/2
  C8/2(xnz) -> [xnz]
  C10/1(yn) -> Delay6/1
  AddSub5/1 -> Delay7/1
  Delay6/1 -> AddSub5/2
  C9/1(yn) -> AddSub5/1
  C8/1(yn) -> Delay4/1
  C7/1(yn) -> AddSub3/1
  AddSub3/1 -> Delay5/1
  Delay4/1 -> AddSub3/2
  C7/2(xnz) -> C8/1(xn)
  C6/1(yn) -> Delay1/1
  AddSub1/1 -> Delay2/1
  Delay1/1 -> AddSub1/2
  C5/1(yn) -> AddSub1/1
  C1/1(yn) -> AddSub/1
  Delay3/1 -> AddSub/2
  AddSub12/1 -> Delay14/1
  Delay13/1 -> AddSub12/2
  C12/2(xnz) -> C13/1(xn)
  C11/2(xnz) -> C12/1(xn)
  C10/2(xnz) -> C11/1(xn)
  C9/2(xnz) -> C10/1(xn)
  [xnz] -> C9/1(xn)
  C6/2(xnz) -> C7/1(xn)
  C5/2(xnz) -> C6/1(xn)
  C4/1(yn) -> Delay13/1
  C4/2(xnz) -> C5/1(xn)
  C3/1(yn) -> AddSub12/1
  C3/2(xnz) -> C4/1(xn)
  C2/2(xnz) -> C3/1(xn)
  FSM/3(load) -> C12/4(LD), C13/4(LD), C14/4(LD), C16/4(LD), C15/4(LD), C11/4(LD), C10/4(LD), C9/4(LD), C1/4(LD), C8/4(LD), C7/4(LD), C6/4(LD), C5/4(LD), C4/4(LD), C3/4(LD), C2/4(LD)
  FSM/2(Coef Addr) -> C12/3(CAddr), C13/3(CAddr), C14/3(CAddr), C16/3(CAddr), C15/3(CAddr), C11/3(CAddr), C10/3(CAddr), C9/3(CAddr), C1/3(CAddr), C8/3(CAddr), C7/3(CAddr), C6/3(CAddr), C5/3(CAddr), C4/3(CAddr), C3/3(CAddr), C2/3(CAddr)
  FSM/1(Data Addr) -> C12/2(DAddr), C13/2(DAddr), C14/2(DAddr), C16/2(DAddr), C15/2(DAddr), C11/2(DAddr), C10/2(DAddr), C9/2(DAddr), C1/2(DAddr), C8/2(DAddr), C7/2(DAddr), C6/2(DAddr), C5/2(DAddr), C4/2(DAddr), C3/2(DAddr), C2/2(DAddr)
  C1/2(xnz) -> C2/1(xn)
  C2/1(yn) -> Delay3/1
  x/1 -> C1/1(xn)
  C13/2(xnz) -> C14/1(xn)
  C14/2(xnz) -> C15/1(xn)
  C15/2(xnz) -> C16/1(xn)
  C15/1(yn) -> AddSub11/1
  C14/1(yn) -> Delay12/1
  C16/1(yn) -> Delay15/1
  Delay12/1 -> AddSub10/2
  Delay15/1 -> AddSub11/2
  AddSub10/1 -> Delay10/1
  AddSub11/1 -> Delay11/1
  Delay10/1 -> AddSub14/1
  Delay11/1 -> AddSub14/2
  AddSub14/1 -> AddSub9/2, Gateway Out6/1
  Gateway Out6/1 -> Long Corr Scope2/1
  Gateway Out7/1 -> Long Corr Scope2/2
  Gateway Out8/1 -> Long Corr Scope2/3

== system ofdm_txrx_supermimo/OFDM Rx MIMO/Packet_Detection/Internal PktDet (Sim Only!) ==
  note: Delay to match latencies of correlation &
      | energy calculation branches
  note: This block is used to detect the start of a packet. It correlates the energy in the received signal in order to
      | determine the packet start.  The signal will be detected in the preamble  (Usually during the third short symbol).
  note: Pkt Det goes high sometime
      | during the third short symbol
  note: Latency = 2
  note: Latency = 3
  note: Latency = 4
  note: Latency = 2
  note: Latency = 1
  AntA I : Inport  OutDataType=sfix(16)  OutScaling=2^0
  AntA Q : Inport  Port=2  OutDataType=sfix(16)  OutScaling=2^0
  A*conj(B) 4xCLK : Subsystem
  Coarse Pkt Det : Scope  NumInputPorts=6
      settings: YMin=-0.03~-1~-1~-1~-1~-5  YMax=0.04~1~1~1~1~5  SaveName=ScopeData28
  Coarse Pkt Det1 : Scope  NumInputPorts=6
      settings: YMin=-0.03~-1~-1~-1~-1~-5  YMax=0.04~1~1~1~1~5  SaveName=ScopeData77
  Convert : xbsIndex_r4/Convert  arith_type="Signed  (2's comp)"  n_bits=18  bin_pt=11  quantization=Truncate  overflow=Flag as error  latency=0  dbl_ovrd=off  pipeline=off  block_type=convert
      settings: xl_area=[0 0 0 0 0 0 0]  block_version=VER_STRING_GOES_HERE
  Delay : xbsIndex_r4/Delay  en=off  latency=16  dbl_ovrd=off  reg_retiming=off  block_type=delay
      settings: infoedit=Hardware notes: A delay line is a chain, each link of which is an SRL16 followed by a flip-flop. If register retiming is enabled, the delay line is a chain of flip-flops.  xl_area=[7 14 0 14 0 0 0]  block_version=VER_STRING_GOES_HERE
  Delay1 : xbsIndex_r4/Delay  en=off  latency=16  dbl_ovrd=off  reg_retiming=off  block_type=delay
      settings: infoedit=Hardware notes: A delay line is a chain, each link of which is an SRL16 followed by a flip-flop. If register retiming is enabled, the delay line is a chain of flip-flops.  xl_area=[7 14 0 14 0 0 0]  block_version=VER_STRING_GOES_HERE
  Delay2 : xbsIndex_r4/Delay  Orientation=down  en=off  latency=3  dbl_ovrd=off  reg_retiming=off  block_type=delay
      settings: infoedit=Hardware notes: A delay line is a chain, each link of which is an SRL16 followed by a flip-flop. If register retiming is enabled, the delay line is a chain of flip-flops.  xl_area=[7 14 0 14 0 0 0]  block_version=VER_STRING_GOES_HERE
  Disregard Subsystem : xbsIndex_r4/Disregard Subsystem  Tag=discardX  block_type=disregard
      settings: block_version=10.1.3
  Down Sample : xbsIndex_r4/Down Sample  sample_ratio=2  sample_phase="Last Value of Frame  (most efficient)"  en=off  latency=1  dbl_ovrd=off  block_type=dsamp
      settings: block_version=VER_STRING_GOES_HERE
  Inverter1 : xbsIndex_r4/Inverter  en=off  latency=0  dbl_ovrd=off  block_type=inv
      settings: xl_area=[0 0 0 0 0 0 0]  block_version=VER_STRING_GOES_HERE
  Logical : xbsIndex_r4/Logical  logical_function=AND  inputs=2  en=off  latency=0  precision=Full  arith_type=Unsigned  n_bits=8  bin_pt=2  align_bp=on  dbl_ovrd=off  block_type=logical
      settings: xl_area=[1 0 0 1 0 0 0]  block_version=VER_STRING_GOES_HERE
  Logical1 : xbsIndex_r4/Logical  logical_function=AND  inputs=2  en=off  latency=0  precision=Full  arith_type=Unsigned  n_bits=8  bin_pt=2  align_bp=on  dbl_ovrd=off  block_type=logical
      settings: xl_area=[1 0 0 1 0 0 0]  block_version=VER_STRING_GOES_HERE
  Magnitude Squared : Subsystem  mask{prec=22, bp=14, multLatency=3, addLatency=1}
      mask prompts: prec "Precision"; bp "Binary Point"; multLatency "Multiplier Latency"; addLatency "Adder Latency"
  Magnitude Squared1 : Subsystem  mask{prec=17, bp=14, multLatency=2, addLatency=0}
      mask prompts: prec "Precision"; bp "Binary Point"; multLatency "Multiplier Latency"; addLatency "Adder Latency"
  Mult1 : xbsIndex_r4/Mult  precision=User Defined  arith_type=Unsigned  n_bits=18  bin_pt=15  quantization=Truncate  overflow=Saturate  en=off  latency=2  dbl_ovrd=off  use_behavioral_HDL=off  use_embedded=on  opt=Speed  optimum_pipeline=off  pipeline=on  use_rpm=off  placement_style=Rectangular shape  block_type=mult
      settings: infoedit=Hardware notes: To use the internal pipeline stage of the dedicated multiplier you must select 'Pipeline for maximum performance'.  xl_area=[22 24 0 44 0 1 0]  block_version=VER_STRING_GOES_HERE
  Mult2 : xbsIndex_r4/Mult  precision=User Defined  arith_type=Unsigned  n_bits=22  bin_pt=14  quantization=Truncate  overflow=Saturate  en=off  latency=2  dbl_ovrd=off  use_behavioral_HDL=off  use_embedded=on  opt=Speed  optimum_pipeline=off  pipeline=on  use_rpm=off  placement_style=Rectangular shape  block_type=mult
      settings: infoedit=Hardware notes: To use the internal pipeline stage of the dedicated multiplier you must select 'Pipeline for maximum performance'.  xl_area=[19 30 0 38 0 2 0]  block_version=VER_STRING_GOES_HERE
  Relational : xbsIndex_r4/Relational  mode=a>b  en=off  latency=1  dbl_ovrd=off  block_type=relational
      settings: xl_area=[11 1 0 22 0 0 0]  block_version=VER_STRING_GOES_HERE
  Relational1 : xbsIndex_r4/Relational  mode=a>b  en=off  latency=3  dbl_ovrd=off  block_type=relational
      settings: xl_area=[10 2 0 19 0 0 0]  block_version=VER_STRING_GOES_HERE
  Slice1 : xbsIndex_r4/Slice  nbits=12  boolean_output=off  mode=Lower Bit Location + Width  bit1=0  base1=MSB of Input  bit0=8  base0=LSB of Input  dbl_ovrd=off  block_type=slice
      settings: infoedit="Extracts a given range of bits from each input sample and presents it at the output.  The output type is ordinarily unsigned with binary point at zero, but can be Boolean when the slice is one bit wide.<P><P>Hardware notes: In hardware this block costs nothing."  block_version=VER_STRING_GOES_HERE
  Slice25 : xbsIndex_r4/Slice  nbits=8  boolean_output=off  mode=Lower Bit Location + Width  bit1=0  base1=MSB of Input  bit0=0  base0=LSB of Input  dbl_ovrd=off  block_type=slice
      settings: infoedit="Extracts a given range of bits from each input sample and presents it at the output.  The output type is ordinarily unsigned with binary point at zero, but can be Boolean when the slice is one bit wide.<P><P>Hardware notes: In hardware this block costs nothing."  block_version=VER_STRING_GOES_HERE
  Sliding Window : Subsystem
  Sliding Window1 : Subsystem
  Sliding Window2 : Subsystem
  To_UFix12_5 : xbsIndex_r4/Reinterpret  force_arith_type=on  arith_type=Unsigned  force_bin_pt=on  bin_pt=5  block_type=cast
      settings: infoedit="Changes type of samples without altering their binary representation.<P><P>Hardware notes: In hardware this block costs nothing.<P><P>Example:  Suppose the input is 6 bits wide, signed, with 2 fractional bits, and the output is forced to unsigned with 0 fractional bits.  Then an input of -2.0 (1110.00 in binary 2's complement) becomes an output of 56 (111000 in binary)."  block_version=VER_STRING_GOES_HERE
  To_UFix8_7 : xbsIndex_r4/Reinterpret  force_arith_type=on  arith_type=Unsigned  force_bin_pt=on  bin_pt=7  block_type=cast
      settings: infoedit="Changes type of samples without altering their binary representation.<P><P>Hardware notes: In hardware this block costs nothing.<P><P>Example:  Suppose the input is 6 bits wide, signed, with 2 fractional bits, and the output is forced to unsigned with 0 fractional bits.  Then an input of -2.0 (1110.00 in binary 2's complement) becomes an output of 56 (111000 in binary)."  block_version=VER_STRING_GOES_HERE
  done{1..12} (12 alike) : xbsIndex_r4/Gateway Out  hdl_port=off  timing_constraint=None  locs_specified=off  LOCs={}  block_type=gatewayout
      settings: infoedit="Gateway out block.  Converts Xilinx fixed point inputs into ouputs of type Simulink integer, double, or fixed point.<P><P>Hardware notes:  In hardware these blocks become top level output ports or are discarded, depending on how they are configured."  xl_area=[0 0 0 0 1 0 0]  block_version=VER_STRING_GOES_HERE
  PktDet : Outport
  wires:
  done6/1 -> Coarse Pkt Det/5
  done3/1 -> Coarse Pkt Det/4
  done5/1 -> Coarse Pkt Det/3
  done4/1 -> Coarse Pkt Det/1
  done1/1 -> Coarse Pkt Det/6
  Down Sample/1 -> Inverter1/1
  Delay2/1 -> done5/1, Relational/1
  Logical/1 -> done6/1, Logical1/1
  Logical1/1 -> done1/1, PktDet/1
  Inverter1/1 -> Logical1/2
  [rx_global_reset] -> Down Sample/1
  Relational1/1 -> Logical/2
  Convert/1 -> Mult1/1, Mult1/2, done12/1
  Relational/1 -> Logical/1
  Magnitude Squared/1(Mag) -> Delay2/1
  Mult2/1 -> Relational/2
  Mult1/1 -> Mult2/1, done3/1, Relational1/1, done7/1
  Sliding Window2/1(Out) -> Convert/1, done9/1
  Magnitude Squared1/1(Mag) -> Sliding Window2/1(In), done11/1
  Sliding Window1/1(Out) -> Magnitude Squared/2(Q)
  Sliding Window/1(Out) -> Magnitude Squared/1(I)
  A*conj(B) 4xCLK/2(Im1) -> Sliding Window1/1(In)
  A*conj(B) 4xCLK/1(Re) -> Sliding Window/1(In)
  Delay1/1 -> A*conj(B) 4xCLK/4(Im[B]), Magnitude Squared1/2(Q), done8/1
  Delay/1 -> A*conj(B) 4xCLK/2(Re[B]), Magnitude Squared1/1(I), done10/1
  AntA I/1 -> done4/1, A*conj(B) 4xCLK/1(Re[A]), Delay/1
  AntA Q/1 -> A*conj(B) 4xCLK/3(Im[A]), Delay1/1, done2/1
  Slice1/1 -> To_UFix12_5/1
  [Rx_IntPktDet_Thresholds] -> Slice25/1, Slice1/1
  Slice25/1 -> To_UFix8_7/1
  To_UFix8_7/1 -> Mult2/2
  To_UFix12_5/1 -> Relational1/2
  done2/1 -> Coarse Pkt Det/2
  done12/1 -> Coarse Pkt Det1/5
  done9/1 -> Coarse Pkt Det1/4
  done11/1 -> Coarse Pkt Det1/3
  done10/1 -> Coarse Pkt Det1/1
  done7/1 -> Coarse Pkt Det1/6
  done8/1 -> Coarse Pkt Det1/2
